simulink model "ClimaCenCfgMgr"  (R2022b)
settings: Solver=FixedStepDiscrete  StartTime=0.0  StopTime=10.0  FixedStep=0.1  RelTol=1e-3
(part 1 of 2)
settings Model: Creator=blue.ge  LastModifiedBy=blue.ge
settings DataIOCC: SaveFormat=StructureWithTime
settings OptimizationCC: DefaultParameterBehavior=Inlined  EnhancedBackFolding=on  PassReuseOutputArgsAs=Individual arguments  ZeroExternalMemoryAtStartup=off  ZeroInternalMemoryAtStartup=off  OptimizeBlockOrder=speed  DifferentSizesBufferReuse=on  OptimizationCustomize=off  EfficientTunableParamExpr=on
settings DebuggingCC: ParameterTunabilityLossMsg=error
settings ModelReferenceCC: ModelReferenceNumInstancesAllowed=Single
settings SFSimCC: MATLABDynamicMemAlloc=off
settings RTWCC: Description=AUTOSAR  SystemTargetFile=autosar.tlc  GenCodeOnly=on  TemplateMakefile=ert_default_tmf
settings CodeAppCC: ForceParamTrailComments=on  IgnoreCustomStorageClasses=off  OperatorAnnotations=on  SimulinkDataObjDesc=on  SFDataObjDesc=on  InsertBlockDesc=on
settings STFCustomTargetCC: TargetLangStandard=C99 (ISO)  MultiwordLength=256  ParMdlRefBuildCompliant=on  CompOptLevelCompliant=on  IncludeMdlTerminateFcn=off  CombineOutputUpdateFcns=on  SuppressErrorStatus=on  ERTCustomFileBanners=on  SupportNonFinite=off  SupportComplex=off  SupportContinuousTime=off  SupportNonInlinedSFcns=off  RemoveResetFunc=on  MATLABClassNameForMDSCustomization=Simulink.SoftwareTarget.ERTCustomization  AutosarCompliant=on  UseToolchainInfoCompliant=on  LookupTableObjectStructAxisOrder=2,1,3,4,...  LUTObjectStructOrderEvenSpacing=Size,Table,Breakpoints  SystemTargetFile=autosar.tlc
settings ERTTargetCC: TargetLangStandard=C99 (ISO)  ConcurrentExecutionCompliant=off  IncludeMdlTerminateFcn=off  SuppressErrorStatus=on  SupportNonFinite=off  SupportComplex=off  ModelStepFunctionPrototypeControlCompliant=off  CPPClassGenCompliant=off  AutosarCompliant=on  LookupTableObjectStructAxisOrder=2,1,3,4,...  LUTObjectStructOrderEvenSpacing=Size,Table,Breakpoints  InlinedParameterPlacement=NonHierarchical  ERTCustomFileTemplate=example_file_process.tlc

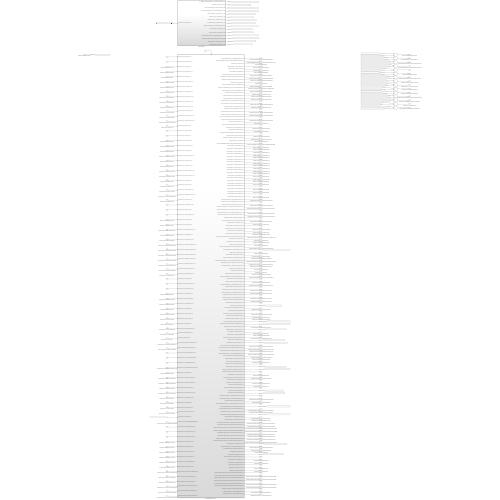
[thumbnail: Simulink's stored preview of the top-level diagram]
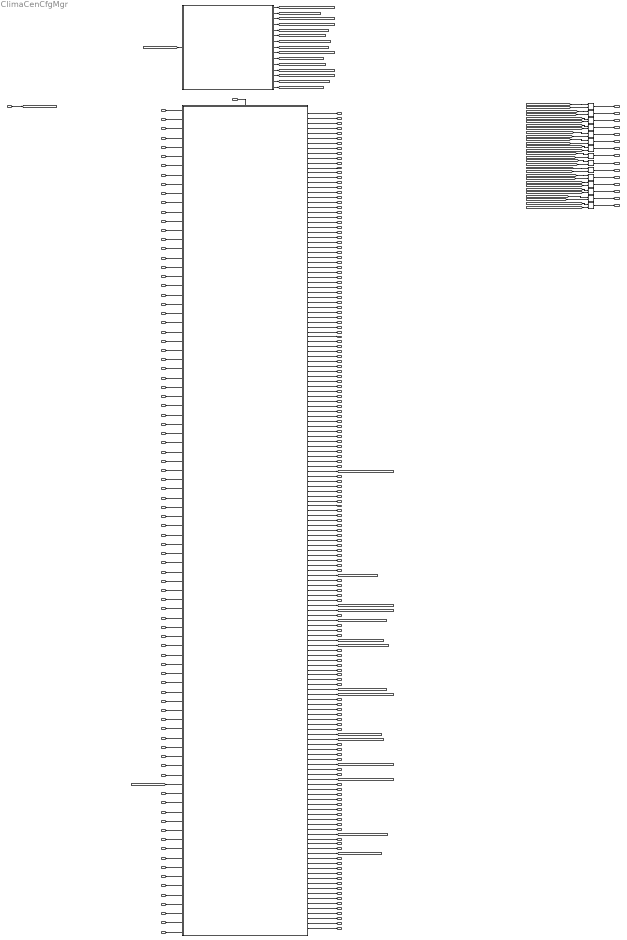
== system ClimaCenCfgMgr ==
  ClimaCenCfgMgr_Runnable : Inport  SampleTime=0.1  OutputFunctionCall=on
  CarCfgForClimaCtrl_CarCfgForRFKEY : Inport  Port=2  OutDataTypeStr=UInt8  PortDimensions=1
  CarCfgForClimaCtrl_CarCfgForAirClng : Inport  Port=3  OutDataTypeStr=Enum: CarCfgForAirClng  PortDimensions=1
  CarCfgForClimaCtrl_CarCfgForAirClnrAdv : Inport  Port=4  OutDataTypeStr=UInt8  PortDimensions=1
  CarCfgForClimaCtrl_CarCfgForAirFrag : Inport  Port=5  OutDataTypeStr=Enum: CarCfgForAirFrag  PortDimensions=1
  CarCfgForClimaCtrl_CarCfgForAirMtrlSnsr : Inport  Port=6  OutDataTypeStr=Enum: CarCfgForAirMtrlSnsrGly  PortDimensions=1
  CarCfgForClimaCtrl_CarCfgForAirQly : Inport  Port=7  OutDataTypeStr=Enum: CarCfgForAirQlyGly  PortDimensions=1
  CarCfgForClimaCtrl_CarCfgForAmntOfSeat : Inport  Port=8  OutDataTypeStr=Enum: CarCfgForAmntOfSeatTyp3  PortDimensions=1
  CarCfgForClimaCtrl_CarCfgForAutDemst : Inport  Port=9  OutDataTypeStr=UInt8  PortDimensions=1
  CarCfgForClimaCtrl_CarCfgForAuxWtrPmp : Inport  Port=10  OutDataTypeStr=Enum: CarCfgForAuxWtrPmp  PortDimensions=1
  CarCfgForClimaCtrl_CarCfgForBypVlvInHvac : Inport  Port=11  OutDataTypeStr=Enum: CarCfgForBypVlvInHvac2  PortDimensions=1
  CarCfgForClimaCtrl_CarCfgForClsVlvInHvac : Inport  Port=12  OutDataTypeStr=Enum: CarCfgForClsVlvInHvac  PortDimensions=1
  CarCfgForClimaCtrl_CarCfgForCmprAcTyp : Inport  Port=13  OutDataTypeStr=UInt8  PortDimensions=1
  CarCfgForClimaCtrl_CarCfgForCmptmtClng : Inport  Port=14  OutDataTypeStr=Enum: CarCfgForCmptmtClng  PortDimensions=1
  CarCfgForClimaCtrl_CarCfgForElecVentAdjmt : Inport  Port=15  OutDataTypeStr=Enum: CarCfgForElecVentAdjmt  PortDimensions=1
  CarCfgForClimaCtrl_CarCfgForExtVentnClimaCtrl : Inport  Port=16  OutDataTypeStr=Enum: CarCfgForExtVentnClimaCtrl  PortDimensions=1
  CarCfgForClimaCtrl_CarCfgForFlcTyp : Inport  Port=17  OutDataTypeStr=Enum: CarCfgForFlcTyp  PortDimensions=1
  CarCfgForClimaCtrl_CarCfgForFoldCSD : Inport  Port=18  OutDataTypeStr=Enum: CarCfgForFoldCSD  PortDimensions=1
  CarCfgForClimaCtrl_CarCfgForFuTyp : Inport  Port=19  OutDataTypeStr=Enum: CarCfgForFuTyp  PortDimensions=1
  CarCfgForClimaCtrl_CarCfgForGlassTyp : Inport  Port=20  OutDataTypeStr=Enum: CarCfgForGlassTyp  PortDimensions=1
  CarCfgForClimaCtrl_CarCfgForHeadRadar : Inport  Port=21  OutDataTypeStr=Enum: HeadRadar  PortDimensions=1
  CarCfgForClimaCtrl_CarCfgForHeatPmp : Inport  Port=22  OutDataTypeStr=Enum: CarCfgForHeatPmpTyp  PortDimensions=1
  CarCfgForClimaCtrl_CarCfgForHeatrDurgDrvg : Inport  Port=23  OutDataTypeStr=Enum: CarCfgForHeatrDurgDrvg  PortDimensions=1
  CarCfgForClimaCtrl_CarCfgForHeatrTyp : Inport  Port=24  OutDataTypeStr=UInt8  PortDimensions=1
  CarCfgForClimaCtrl_CarCfgForHvacFilTyp : Inport  Port=25  OutDataTypeStr=Enum: CarCfgForHvacFilTypGly  PortDimensions=1
  CarCfgForClimaCtrl_CarCfgForHvacFlrSnsrForT : Inport  Port=26  OutDataTypeStr=Enum: CarCfgForHvacFlrSnsrForT  PortDimensions=1
  CarCfgForClimaCtrl_CarCfgForHvacHeatrSnsrT : Inport  Port=27  OutDataTypeStr=Enum: CarCfgForHvacHeatrSnsrT  PortDimensions=1
  CarCfgForClimaCtrl_CarCfgForHvacRe : Inport  Port=28  OutDataTypeStr=UInt8  PortDimensions=1
  CarCfgForClimaCtrl_CarCfgForHvacTyp : Inport  Port=29  OutDataTypeStr=UInt8  PortDimensions=1
  CarCfgForClimaCtrl_CarCfgForLoVoltElecCfg : Inport  Port=30  OutDataTypeStr=Enum: CarCfgForLoVoltElecCfg  PortDimensions=1
  CarCfgForClimaCtrl_CarCfgForNavSysandIntnmap : Inport  Port=31  OutDataTypeStr=Enum: CarCfgForNavSysandIntnmap  PortDimensions=1
  CarCfgForClimaCtrl_CarCfgForNrOfDoor : Inport  Port=32  OutDataTypeStr=Enum: CarCfgForNrOfDoor2  PortDimensions=1
  CarCfgForClimaCtrl_CarCfgForPipeInFuTank : Inport  Port=33  OutDataTypeStr=Enum: CarCfgForPipeInFuTank  PortDimensions=1
  CarCfgForClimaCtrl_CarCfgForPreClng : Inport  Port=34  OutDataTypeStr=Enum: CarCfgForPreClng  PortDimensions=1
  CarCfgForClimaCtrl_CarCfgForPrkgCDSNotAvl : Inport  Port=35  OutDataTypeStr=Enum: CarCfgForPrkgCDSNotAvl  PortDimensions=1
  CarCfgForClimaCtrl_CarCfgForPrkgClima : Inport  Port=36  OutDataTypeStr=Enum: CarCfgForPrkgClima  PortDimensions=1
  CarCfgForClimaCtrl_CarCfgForPrpsnTyp : Inport  Port=37  OutDataTypeStr=Enum: CarCfgForPrpsnTypGly  PortDimensions=1
  CarCfgForClimaCtrl_CarCfgForRemEngStrtPreCdn : Inport  Port=38  OutDataTypeStr=Enum: CarCfgForRemEngStrtPreCdn  PortDimensions=1
  CarCfgForClimaCtrl_CarCfgForRoofHatch : Inport  Port=39  OutDataTypeStr=Enum: CarCfgForRoofHatch  PortDimensions=1
  CarCfgForClimaCtrl_CarCfgForSeatHeatgCal : Inport  Port=40  OutDataTypeStr=Enum: CarCfgForSeatHeatgCal1  PortDimensions=1
  CarCfgForClimaCtrl_CarCfgForSeatHeatgForRowFirst : Inport  Port=41  OutDataTypeStr=Enum: CarCfgForSeatHeatgForRowFirst  PortDimensions=1
  CarCfgForClimaCtrl_CarCfgForSeatHeatgForRowSec : Inport  Port=42  OutDataTypeStr=Enum: CarCfgForSeatHeatgForRowSec  PortDimensions=1
  CarCfgForClimaCtrl_CarCfgForSeatVentnForRowFirst : Inport  Port=43  OutDataTypeStr=Enum: CarCfgForSeatVentnForRowFirst1  PortDimensions=1
  CarCfgForClimaCtrl_CarCfgForSeatVentnForRowSec : Inport  Port=44  OutDataTypeStr=Enum: CarCfgForSeatVentnForRowSecGly  PortDimensions=1
  CarCfgForClimaCtrl_CarCfgForSecRowVentnCenter : Inport  Port=45  OutDataTypeStr=Enum: CarCfgForSecRowVentnCenter  PortDimensions=1
  CarCfgForClimaCtrl_CarCfgForSecRowVentnSide : Inport  Port=46  OutDataTypeStr=Enum: CarCfgForSecRowVentnSide  PortDimensions=1
  CarCfgForClimaCtrl_CarCfgForSmallFlowCyc : Inport  Port=47  OutDataTypeStr=Enum: CarCfgForSmallFlowCyc  PortDimensions=1
  CarCfgForClimaCtrl_CarCfgForSmkSnsr : Inport  Port=48  OutDataTypeStr=Enum: CarCfgForSmkSnsr  PortDimensions=1
  CarCfgForClimaCtrl_CarCfgForSnsrForCmptmtT : Inport  Port=49  OutDataTypeStr=Enum: CarCfgForSnsrForCmptmtT  PortDimensions=1
  CarCfgForClimaCtrl_CarCfgForSnsrHumPrsnt : Inport  Port=50  OutDataTypeStr=Enum: CarCfgForSnsrHumPrsnt  PortDimensions=1
  CarCfgForClimaCtrl_CarCfgForStartDevice : Inport  Port=51  OutDataTypeStr=Enum: StartDevice  PortDimensions=1
  CarCfgForClimaCtrl_CarCfgForSteerWhlHeatg : Inport  Port=52  OutDataTypeStr=UInt8  PortDimensions=1
  CarCfgForClimaCtrl_CarCfgForSteerWhlPosn : Inport  Port=53  OutDataTypeStr=Enum: CarCfgForSteerWhlPosn  PortDimensions=1
  CarCfgForClimaCtrl_CarCfgForSunSnsr : Inport  Port=54  OutDataTypeStr=Enum: CarCfgForSunSnsr  PortDimensions=1
  CarCfgForClimaCtrl_CarCfgForTelmNodTyp : Inport  Port=55  OutDataTypeStr=Enum: CarCfgForTelmNodTyp  PortDimensions=1
  CarCfgForClimaCtrl_CarCfgForThermMngt : Inport  Port=56  OutDataTypeStr=Enum: CarCfgForThermMngt  PortDimensions=1
  CarCfgForClimaCtrl_CarCfgForVehTyp : Inport  Port=57  OutDataTypeStr=Enum: CarCfgForVehTyp3  PortDimensions=1
  CarCfgForClimaCtrl_CarCfgForVgaMotorExistSts : Inport  Port=58  OutDataTypeStr=CarCfgForVgaMotorExistSts  PortDimensions=1
  CarCfgForClimaCtrl_CarCfgForWindHeatd : Inport  Port=59  OutDataTypeStr=Enum: CarCfgForWindHeatd  PortDimensions=1
  CooltVlvOutpTyp_CooltVlvOutpTyp : Inport  Port=60  OutDataTypeStr=Enum: CooltVlvOutpTyp  PortDimensions=1
  HvacCfgForClimaCtrl_HvacCfgForHvacFrontBlowerType : Inport  Port=61  OutDataTypeStr=HvacCfgForHvacFrontBlowerType  PortDimensions=1
  HvacCfgForClimaCtrl_HvacCfgForHvacMiscExistSts : Inport  Port=62  OutDataTypeStr=HvacCfgForHvacMiscExistSts  PortDimensions=1
  HvacCfgForClimaCtrl_HvacCfgForHvacMotorExistSts : Inport  Port=63  OutDataTypeStr=HvacCfgForHvacMotorExistSts  PortDimensions=1
  HvacCfgForClimaCtrl_HvacCfgForHvacSensorExistSts : Inport  Port=64  OutDataTypeStr=HvacCfgForHvacSensorExistSts  PortDimensions=1
  CarCfgForClimaCtrl_CarCfgFor3RDROWSEATHEAT : Inport  Port=65  OutDataTypeStr=UInt8  PortDimensions=1
  CarCfgForClimaCtrl_CarCfgFor3RDROWSEATVENTILATED : Inport  Port=66  OutDataTypeStr=UInt8  PortDimensions=1
  CarCfgForClimaCtrl_CarCfgForBRAND : Inport  Port=67  OutDataTypeStr=UInt8  PortDimensions=1
  CarCfgForClimaCtrl_CarCfgForCabinOxgenSupply : Inport  Port=68  OutDataTypeStr=UInt8  PortDimensions=1
  CarCfgForClimaCtrl_CarCfgForElectricLiftingModule : Inport  Port=69  OutDataTypeStr=UInt8  PortDimensions=1
  CarCfgForClimaCtrl_CarCfgForFloatingDriving : Inport  Port=70  OutDataTypeStr=UInt8  PortDimensions=1
  CarCfgForClimaCtrl_CarCfgForInteriorViewCamera : Inport  Port=71  OutDataTypeStr=Enum: CarCfgForInteriorViewCamera  PortDimensions=1
  CarCfgForClimaCtrl_CarCfgForLifeDetection : Inport  Port=72  OutDataTypeStr=Enum: LifeDetection  PortDimensions=1
  CarCfgForClimaCtrl_CarCfgForMARKET : Inport  Port=73  OutDataTypeStr=UInt8  PortDimensions=1
  CarCfgForClimaCtrl_CarCfgForPowerSeats : Inport  Port=74  OutDataTypeStr=Enum: CarCfgForPowerSeats  PortDimensions=1
  CarCfgForClimaCtrl_CarCfgForPTCInFrontHVAC : Inport  Port=75  OutDataTypeStr=UInt8  PortDimensions=1
  CarCfgForClimaCtrl_CarCfgForTEMPEREDSTORAGEBOX : Inport  Port=76  OutDataTypeStr=UInt8  PortDimensions=1
  CarCfgForClimaCtrl_CarCfgForVentLiftMotorExiSts : Inport  Port=77  OutDataTypeStr=UInt32  PortDimensions=1
  CarCfgForClimaCtrl_CarCfgForWadingAssistSystem : Inport  Port=78  OutDataTypeStr=UInt8  PortDimensions=1
  CarCfgForClimaCtrl_CarCfgWindFollowHumanMov : Inport  Port=79  OutDataTypeStr=UInt8  PortDimensions=1
  CenCfgMgrForCompile_CarCfgForAirClnrAdv : Inport  Port=80  OutDataTypeStr=Enum: CarCfgForAirClnrAdv  PortDimensions=1
  CenCfgMgrForCompile_CarCfgForAutDemst : Inport  Port=81  OutDataTypeStr=Enum: CarCfgForAutDemst  PortDimensions=1
  CenCfgMgrForCompile_CarCfgForCmprAcTyp3 : Inport  Port=82  OutDataTypeStr=Enum: CarCfgForCmprAcTyp3  PortDimensions=1
  CenCfgMgrForCompile_CarCfgForHeatrTypGly : Inport  Port=83  OutDataTypeStr=Enum: CarCfgForHeatrTypGly  PortDimensions=1
  CenCfgMgrForCompile_CarCfgForSteerWhlHeatg : Inport  Port=84  OutDataTypeStr=Enum: CarCfgForSteerWhlHeatg  PortDimensions=1
  HvacCfgForClimaCtrl_HvacCfgForAQSTypes : Inport  Port=85  OutDataTypeStr=UInt8  PortDimensions=1
  HvacCfgForClimaCtrl_HvacCfgForHvacLowSideRETXVESts : Inport  Port=86  OutDataTypeStr=UInt8  PortDimensions=1
  HvacCfgForClimaCtrl_HvacCfgForHvacReBlowerType : Inport  Port=87  OutDataTypeStr=UInt8  PortDimensions=1
  HvacCfgForClimaCtrl_HvacCfgForHvacReVentPstn : Inport  Port=88  OutDataTypeStr=UInt8  PortDimensions=1
  HvacCfgForClimaCtrl_HvacCfgForHvacSnsrRCmptmtESts : Inport  Port=89  OutDataTypeStr=UInt8  PortDimensions=1
  HvacCfgForClimaCtrl_HvacCfgForHvacSnsrReHexESts : Inport  Port=90  OutDataTypeStr=UInt8  PortDimensions=1
  HvacCfgReqVersionReplace_HvacCfgReqVersionReplace : Inport  Port=91  OutDataTypeStr=UInt8  PortDimensions=1
  ClimaCenCfgMgr_Init : Subsystem
  ClimaCenCfgMgr_Runnable_sys : Subsystem
  ClimaCfgForAirMtrlSnsrPrsnt1_ClimaCfgForAirMtrlSnsrPrsnt1_merge : Merge
  ClimaCfgForFlcTyp_ClimaCfgForFlcTyp_merge : Merge
  ClimaCfgForForLoVoltElecCfg_ClimaCfgForForLoVoltElecCfg_merge : Merge
  ClimaCfgForForRemEngStrtPreCdn_ClimaCfgForForRemEngStrtPreCdn_merge : Merge
  ClimaCfgForFuHeatrTyp1_ClimaCfgForFuHeatrTyp1_merge : Merge
  ClimaCfgForHeatrTyp1_ClimaCfgForHeatrTyp1_merge : Merge
  ClimaCfgForHvHeatrTyp1_ClimaCfgForHvHeatrTyp1_merge : Merge
  ClimaCfgForHvacFilVrnt1_ClimaCfgForHvacFilVrnt1_merge : Merge
  ClimaCfgForNavSysandIntnmap_ClimaCfgForNavSysandIntnmap_merge : Merge
  ClimaCfgForPrpsnTyp_ClimaCfgForPrpsnTyp_merge : Merge
  ClimaCfgForRoofHatch_ClimaCfgForRoofHatch_merge : Merge
  ClimaCfgForSeatHeatgForRowSec_ClimaCfgForSeatHeatgForRowSec_merge : Merge
  ClimaCfgForSeatVentnForRowSec_ClimaCfgForSeatVentnForRowSec_merge : Merge
  ClimaCfgForStatrtDevice_ClimaCfgForStartDevice_merge : Merge
  ClimaCfgForSunSnsr1_ClimaCfgForSunSnsr1_merge : Merge
  ClimaCfgForAirMtrlSnsrPrsnt1_ClimaCfgForAirMtrlSnsrPrsnt1 : Outport  OutDataTypeStr=Enum: CarCfgForAirMtrlSnsrGly  PortDimensions=1
  ClimaCfgForFlcTyp_ClimaCfgForFlcTyp : Outport  Port=2  OutDataTypeStr=Enum: CarCfgForFlcTyp  PortDimensions=1
  ClimaCfgForForLoVoltElecCfg_ClimaCfgForForLoVoltElecCfg : Outport  Port=3  OutDataTypeStr=Enum: CarCfgForLoVoltElecCfg  PortDimensions=1
  ClimaCfgForForRemEngStrtPreCdn_ClimaCfgForForRemEngStrtPreCdn : Outport  Port=4  OutDataTypeStr=Enum: CarCfgForRemEngStrtPreCdn  PortDimensions=1
  ClimaCfgForFuHeatrTyp1_ClimaCfgForFuHeatrTyp1 : Outport  Port=5  OutDataTypeStr=Enum: ClimaCfgForFuHeatrTyp1  PortDimensions=1
  ClimaCfgForHeatrTyp1_ClimaCfgForHeatrTyp1 : Outport  Port=6  OutDataTypeStr=Enum: ClimaCfgForHeatrTyp1  PortDimensions=1
  ClimaCfgForHvacFilVrnt1_ClimaCfgForHvacFilVrnt1 : Outport  Port=7  OutDataTypeStr=Enum: CarCfgForHvacFilTypGly  PortDimensions=1
  ClimaCfgForHvHeatrTyp1_ClimaCfgForHvHeatrTyp1 : Outport  Port=8  OutDataTypeStr=Enum: ClimaCfgForHvHeatrTyp1  PortDimensions=1
  ClimaCfgForNavSysandIntnmap_ClimaCfgForNavSysandIntnmap : Outport  Port=9  OutDataTypeStr=Enum: CarCfgForNavSysandIntnmap  PortDimensions=1
  ClimaCfgForPrpsnTyp_ClimaCfgForPrpsnTyp : Outport  Port=10  OutDataTypeStr=Enum: CarCfgForPrpsnTypGly  PortDimensions=1
  ClimaCfgForRoofHatch_ClimaCfgForRoofHatch : Outport  Port=11  OutDataTypeStr=Enum: CarCfgForRoofHatch  PortDimensions=1
  ClimaCfgForSeatHeatgForRowSec_ClimaCfgForSeatHeatgForRowSec : Outport  Port=12  OutDataTypeStr=Enum: CarCfgForSeatHeatgForRowSecGly  PortDimensions=1
  ClimaCfgForSeatVentnForRowSec_ClimaCfgForSeatVentnForRowSec : Outport  Port=13  OutDataTypeStr=Enum: CarCfgForSeatVentnForRowSecGly  PortDimensions=1
  ClimaCfgForStatrtDevice_ClimaCfgForStartDevice : Outport  Port=14  OutDataTypeStr=Enum: StartDevice  PortDimensions=1
  ClimaCfgForSunSnsr1_ClimaCfgForSunSnsr1 : Outport  Port=15  OutDataTypeStr=Enum: CarCfgForSunSnsr  PortDimensions=1
  ClimaCfgFor3RDROWSEATHEAT_ClimaCfgFor3RDROWSEATHEAT : Outport  Port=16  OutDataTypeStr=UInt8  PortDimensions=1
  ClimaCfgFor3RDROWSEATVENTILATED_ClimaCfgFor3RDROWSEATVENTILATED : Outport  Port=17  OutDataTypeStr=UInt8  PortDimensions=1
  ClimaCfgForAAC_ClimaCfgForAAC : Outport  Port=18  OutDataTypeStr=Enum: CarCfgForAirClng  PortDimensions=1
  ClimaCfgForAQSTypes_ClimaCfgForAQSTypes : Outport  Port=19  OutDataTypeStr=UInt8  PortDimensions=1
  ClimaCfgForAutDemst_ClimaCfgForAutDemst : Outport  Port=20  OutDataTypeStr=Boolean  PortDimensions=1
  ClimaCfgForBRAND_ClimaCfgForBRAND : Outport  Port=21  OutDataTypeStr=Enum: ClimaCfgForBRAND  PortDimensions=1
  ClimaCfgForCabinOxgenSupply_ClimaCfgForCabinOxgenSupply : Outport  Port=22  OutDataTypeStr=Enum: CabinOxgenSupply  PortDimensions=1
  ClimaCfgForDrvrSeatVentWthSmf2_ClimaCfgForDrvrSeatVentWthSmf2 : Outport  Port=23  OutDataTypeStr=UInt8  PortDimensions=1
  ClimaCfgForElectricLiftingModule_ClimaCfgForElectricLiftingModule : Outport  Port=24  OutDataTypeStr=Enum: ElcTrLFtngMdle  PortDimensions=1
  ClimaCfgForErev1_ClimaCfgForErev1 : Outport  Port=25  OutDataTypeStr=Boolean  PortDimensions=1
  ClimaCfgForFLOATINGDRVING_ClimaCfgForFLOATINGDRVING : Outport  Port=26  OutDataTypeStr=Enum: FLOATINGDRVING  PortDimensions=1
  ClimaCfgForHvacLowSideRETXVESts_ClimaCfgForHvacLowSideRETXVESts : Outport  Port=27  OutDataTypeStr=Enum: ClimaCfgForHvacLowSideRETXVESts  PortDimensions=1
  ClimaCfgForHvacReBlowerType_ClimaCfgForHvacReBlowerType : Outport  Port=28  OutDataTypeStr=Enum: ClimaCfgForHvacReBlowerType  PortDimensions=1
  ClimaCfgForHvacReTmpZn_ClimaCfgForHvacReTmpZn : Outport  Port=29  OutDataTypeStr=UInt8  PortDimensions=1
  ClimaCfgForHvacReVentPstn_ClimaCfgForRowSecAtFlrLeRe : Outport  Port=30  OutDataTypeStr=UInt8  PortDimensions=1
  ClimaCfgForHvacReVentPstn_ClimaCfgForRowSecAtFlrRiRe : Outport  Port=31  OutDataTypeStr=UInt8  PortDimensions=1
  ClimaCfgForHvacReVentPstn_ClimaCfgForRowSecAtVentSideTopLe : Outport  Port=32  OutDataTypeStr=UInt8  PortDimensions=1
  ClimaCfgForHvacReVentPstn_ClimaCfgForRowSecAtVentSideTopRi : Outport  Port=33  OutDataTypeStr=UInt8  PortDimensions=1
  ClimaCfgForHvacReVentPstn_ClimaCfgForRowThrdAtFlrLe : Outport  Port=34  OutDataTypeStr=UInt8  PortDimensions=1
  ClimaCfgForHvacReVentPstn_ClimaCfgForRowThrdAtFlrRi : Outport  Port=35  OutDataTypeStr=UInt8  PortDimensions=1
  ClimaCfgForHvacReVentPstn_ClimaCfgForRowThrdAtVentSideTopLe : Outport  Port=36  OutDataTypeStr=UInt8  PortDimensions=1
  ClimaCfgForHvacReVentPstn_ClimaCfgForRowThrdAtVentSideTopRi : Outport  Port=37  OutDataTypeStr=UInt8  PortDimensions=1
  ClimaCfgForHvacSnsrRCmptmtESts_ClimaCfgForHvacSnsrRCmptmtESts : Outport  Port=38  OutDataTypeStr=Enum: ClimaCfgForHvacSnsrRCmptmtESts  PortDimensions=1
  ClimaCfgForHvacSnsrReHexESts_ClimaCfgForHvacSnsrReHexESts : Outport  Port=39  OutDataTypeStr=Enum: ClimaCfgForHvacSnsrReHexESts  PortDimensions=1
  ClimaCfgForHvacTyp_ClimaCfgForHvacTyp : Outport  Port=40  OutDataTypeStr=Enum: CarCfgForHvacTyp2  PortDimensions=1
  ClimaCfgForInteriorViewCamera_ClimaCfgForInteriorViewCamera : Outport  Port=41  OutDataTypeStr=Enum: CarCfgForInteriorViewCamera  PortDimensions=1
  ClimaCfgForLifeDetection_ClimaCfgForLifeDetection : Outport  Port=42  OutDataTypeStr=Enum: LifeDetection  PortDimensions=1
  ClimaCfgForMARKET_ClimaCfgForMARKET : Outport  Port=43  OutDataTypeStr=UInt8  PortDimensions=1
  ClimaCfgForPassSeatVentWthSmf2_ClimaCfgForPassSeatVentWthSmf2 : Outport  Port=44  OutDataTypeStr=UInt8  PortDimensions=1
  ClimaCfgForPowerSeats_ClimaCfgForPowerSeats : Outport  Port=45  OutDataTypeStr=Enum: CarCfgForPowerSeats  PortDimensions=1
  ClimaCfgForPTCInFrontHVAC_ClimaCfgForPTCInFrontHVAC : Outport  Port=46  OutDataTypeStr=Enum: FrontHeaterExchanger  PortDimensions=1
  ClimaCfgForRFKEY_ClimaCfgForRFKEY : Outport  Port=47  OutDataTypeStr=Enum: RFKEY  PortDimensions=1
  ClimaCfgForTEMPEREDSTORAGEBOX_ClimaCfgForTEMPEREDSTORAGEBOX : Outport  Port=48  OutDataTypeStr=Enum: TEMPEREDSTORAGEBOX  PortDimensions=1
  ClimaCfgForVgaMotor1_ClimaCfgForVgaMotor1 : Outport  Port=49  OutDataTypeStr=Boolean  PortDimensions=1
  ClimaCfgForVgaMotor10_ClimaCfgForVgaMotor10 : Outport  Port=50  OutDataTypeStr=Boolean  PortDimensions=1
  ClimaCfgForVgaMotor11_ClimaCfgForVgaMotor11 : Outport  Port=51  OutDataTypeStr=Boolean  PortDimensions=1
  ClimaCfgForVgaMotor12_ClimaCfgForVgaMotor12 : Outport  Port=52  OutDataTypeStr=Boolean  PortDimensions=1
  ClimaCfgForVgaMotor13_ClimaCfgForVgaMotor13 : Outport  Port=53  OutDataTypeStr=Boolean  PortDimensions=1
  ClimaCfgForVgaMotor14_ClimaCfgForVgaMotor14 : Outport  Port=54  OutDataTypeStr=Boolean  PortDimensions=1
  ClimaCfgForVgaMotor15_ClimaCfgForVgaMotor15 : Outport  Port=55  OutDataTypeStr=Boolean  PortDimensions=1
  ClimaCfgForVgaMotor16_ClimaCfgForVgaMotor16 : Outport  Port=56  OutDataTypeStr=Boolean  PortDimensions=1
  ClimaCfgForVgaMotor17_ClimaCfgForVgaMotor17 : Outport  Port=57  OutDataTypeStr=Boolean  PortDimensions=1
  ClimaCfgForVgaMotor18_ClimaCfgForVgaMotor18 : Outport  Port=58  OutDataTypeStr=Boolean  PortDimensions=1
  ClimaCfgForVgaMotor19_ClimaCfgForVgaMotor19 : Outport  Port=59  OutDataTypeStr=Boolean  PortDimensions=1
  ClimaCfgForVgaMotor2_ClimaCfgForVgaMotor2 : Outport  Port=60  OutDataTypeStr=Boolean  PortDimensions=1
  ClimaCfgForVgaMotor20_ClimaCfgForVgaMotor20 : Outport  Port=61  OutDataTypeStr=Boolean  PortDimensions=1
  ClimaCfgForVgaMotor3_ClimaCfgForVgaMotor3 : Outport  Port=62  OutDataTypeStr=Boolean  PortDimensions=1
  ClimaCfgForVgaMotor4_ClimaCfgForVgaMotor4 : Outport  Port=63  OutDataTypeStr=Boolean  PortDimensions=1
  ClimaCfgForVgaMotor5_ClimaCfgForVgaMotor5 : Outport  Port=64  OutDataTypeStr=Boolean  PortDimensions=1
  ClimaCfgForVgaMotor6_ClimaCfgForVgaMotor6 : Outport  Port=65  OutDataTypeStr=Boolean  PortDimensions=1
  ClimaCfgForVgaMotor7_ClimaCfgForVgaMotor7 : Outport  Port=66  OutDataTypeStr=Boolean  PortDimensions=1
  ClimaCfgForVgaMotor8_ClimaCfgForVgaMotor8 : Outport  Port=67  OutDataTypeStr=Boolean  PortDimensions=1
  ClimaCfgForVgaMotor9_ClimaCfgForVgaMotor9 : Outport  Port=68  OutDataTypeStr=Boolean  PortDimensions=1
  ClimaCfgForWadingAssistSystem_ClimaCfgForWadingAssistSystem : Outport  Port=69  OutDataTypeStr=Enum: WadingAssistSystem  PortDimensions=1
  ClimaCfgHvacForFrntLeTempActr_ClimaCfgHvacForFrntLeTempActr : Outport  Port=70  OutDataTypeStr=Boolean  PortDimensions=1
  ClimaCfgHvacForFrntRiTempActr_ClimaCfgHvacForFrntRiTempActr : Outport  Port=71  OutDataTypeStr=Boolean  PortDimensions=1
  ClimaCfgHvacFrntForRowSecForActrTLe_ClimaCfgHvacFrntForRowSecForActrTLe : Outport  Port=72  OutDataTypeStr=Boolean  PortDimensions=1
  ClimaCfgHvacFrntForRowSecForActrTRi_ClimaCfgHvacFrntForRowSecForActrTRi : Outport  Port=73  OutDataTypeStr=Boolean  PortDimensions=1
  ClimaCfgHvacReForRowSecForActrTLe_ClimaCfgHvacReForRowSecForActrTLe : Outport  Port=74  OutDataTypeStr=UInt8  PortDimensions=1
  ClimaCfgHvacReForRowSecForActrTRi_ClimaCfgHvacReForRowSecForActrTRi : Outport  Port=75  OutDataTypeStr=UInt8  PortDimensions=1
  ClimaCfgReqVersionReplace_ClimaCfgReqVersionReplace : Outport  Port=76  OutDataTypeStr=UInt8  PortDimensions=1
  ClimaCfgWindFollowHumanMov_ClimaCfgWindFollowHumanMov : Outport  Port=77  OutDataTypeStr=Enum: WndFllwngHmnMvmntFnctn  PortDimensions=1
  ClimaCfgForActrModVrnt1_ClimaCfgForActrInd1 : Outport  Port=78  OutDataTypeStr=Enum: ClimaCfgForActrInd1  PortDimensions=1
  ClimaCfgForActrModVrnt1_ClimaCfgForActrModVrnt1 : Outport  Port=79  OutDataTypeStr=Enum: ClimaCfgForActrModVrnt1  PortDimensions=1
  ClimaCfgForActrModVrnt1_ClimaCfgForActrModVrntCen : Outport  Port=80  OutDataTypeStr=Enum: ClimaCfgForActrModVrntCen  PortDimensions=1
  ClimaCfgForActrModVrnt2_ClimaCfgForActrInd2 : Outport  Port=81  OutDataTypeStr=Enum: ClimaCfgForActrInd2  PortDimensions=1
  ClimaCfgForActrModVrnt2_ClimaCfgForActrModVrnt2 : Outport  Port=82  OutDataTypeStr=Enum: ClimaCfgForActrModVrnt2  PortDimensions=1
  ClimaCfgForActrRecircAndOsaCmnPrsnt1_ClimaCfgForActrRecircAndOsaCmnPrsnt1 : Outport  Port=83  OutDataTypeStr=Enum: ClimaCfgForActrRecircAndOsaCmnPrsnt1  PortDimensions=1
  ClimaCfgForAirClng1_ClimaCfgForAirClng1 : Outport  Port=84  OutDataTypeStr=Boolean  PortDimensions=1
  ClimaCfgForAirClnrAdv1_ClimaCfgForAirClnrAdv1 : Outport  Port=85  OutDataTypeStr=Boolean  PortDimensions=1
  ClimaCfgForAirFrag_ClimaCfgForAirFrag : Outport  Port=86  OutDataTypeStr=Boolean  PortDimensions=1
  ClimaCfgForAirMtrlSnsrAPMAPrsnt1_ClimaCfgForAirMtrlSnsrAPMAPrsnt1 : Outport  Port=87  OutDataTypeStr=Boolean  PortDimensions=1
  ClimaCfgForAirQly1_ClimaCfgForAirQly1 : Outport  Port=88  OutDataTypeStr=Boolean  PortDimensions=1
  ClimaCfgForAmntOfSeat1_ClimaCfgForAmntOfSeat1 : Outport  Port=89  OutDataTypeStr=Enum: ClimaCfgForAmntOfSeat1  PortDimensions=1
  ClimaCfgForAmntOfSeatRow1_ClimaCfgForAmntOfSeatRow1 : Outport  Port=90  OutDataTypeStr=Enum: ClimaCfgForAmntOfSeatRow1  PortDimensions=1
  ClimaCfgForBlowrInRowSecVentSidePrsnt1_ClimaCfgForBlowrInRowSecVentSidePrsnt1 : Outport  Port=91  OutDataTypeStr=Boolean  PortDimensions=1
  ClimaCfgForBypVlvInHvacExtCtrl1_ClimaCfgForBypVlvInHvacExtCtrl1 : Outport  Port=92  OutDataTypeStr=Boolean  PortDimensions=1
  ClimaCfgForBypVlvInHvacPrsnt1_ClimaCfgForBypVlvInHvacPrsnt1 : Outport  Port=93  OutDataTypeStr=Boolean  PortDimensions=1
  ClimaCfgForCarTyp1_ClimaCfgForCarTyp1 : Outport  Port=94  OutDataTypeStr=Enum: ClimaCfgForCarTyp1  PortDimensions=1
  ClimaCfgForCds1_ClimaCfgForCds1 : Outport  Port=95  OutDataTypeStr=Boolean  PortDimensions=1
  ClimaCfgForClimaCtrlMan1_ClimaCfgForClimaCtrlMan1 : Outport  Port=96  OutDataTypeStr=Boolean  PortDimensions=1
  ClimaCfgForClimaCtrlSwtModlPrsnt_ClimaCfgForClimaCtrlSwtModlPrsnt : Outport  Port=97  OutDataTypeStr=Boolean  PortDimensions=1
  ClimaCfgForCmptmtClng_ClimaCfgForCmptmtClng : Outport  Port=98  OutDataTypeStr=Boolean  PortDimensions=1
  ClimaCfgForCmptmtSnsr1_ClimaCfgForCmptmtSnsr1 : Outport  Port=99  OutDataTypeStr=Enum: ClimaCfgForCmptmtSnsr1  PortDimensions=1
  ClimaCfgForComIfGenn2ForTelm1_ClimaCfgForComIfGenn2ForTelm1 : Outport  Port=100  OutDataTypeStr=Boolean  PortDimensions=1
  ClimaCfgForCoolgPreCdn1_ClimaCfgForCoolgPreCdn1 : Outport  Port=101  OutDataTypeStr=Enum: ClimaCfgForCoolgPreCdn1  PortDimensions=1
  ClimaCfgForCooltCircForClima1_ClimaCfgForCooltCircForClima1 : Outport  Port=102  OutDataTypeStr=Boolean  PortDimensions=1
  ClimaCfgForCpilHeatrElecPrsnt1_ClimaCfgForCpilHeatrElecPrsnt1 : Outport  Port=103  OutDataTypeStr=Boolean  PortDimensions=1
  ClimaCfgForEcuCooltVlvOutp1_ClimaCfgForEcuCooltVlvOutp1 : Outport  Port=104  OutDataTypeStr=Enum: ClimaCfgForEcuCooltVlvOutp1  PortDimensions=1
  ClimaCfgForEngCooltPmpElec1_ClimaCfgForEngCooltPmpElec1 : Outport  Port=105  OutDataTypeStr=Boolean  PortDimensions=1
  ClimaCfgForExtVentnClimaCtrl_ClimaCfgForExtVentnClimaCtrl : Outport  Port=106  OutDataTypeStr=Enum: CarCfgForExtVentnClimaCtrl  PortDimensions=1
  ClimaCfgForFanPotiCtrld1_ClimaCfgForFanPotiCtrld1 : Outport  Port=107  OutDataTypeStr=Boolean  PortDimensions=1
  ClimaCfgForFlrSnsrInHvac1_ClimaCfgForFlrSnsrInHvac1 : Outport  Port=108  OutDataTypeStr=Boolean  PortDimensions=1
  ClimaCfgForFoldCSD_ClimaCfgForFoldCSD : Outport  Port=109  OutDataTypeStr=Boolean  PortDimensions=1
  ClimaCfgForForElecVentAdjmt_ClimaCfgForForElecVentAdjmt : Outport  Port=110  OutDataTypeStr=Boolean  PortDimensions=1
  ClimaCfgForForHeatPmp_ClimaCfgForForHeatPmp : Outport  Port=111  OutDataTypeStr=Enum: CarCfgForHeatPmpTyp  PortDimensions=1
  ClimaCfgForForHeatPmpGly_ClimaCfgForForHeatPmp : Outport  Port=112  OutDataTypeStr=Enum: CarCfgForHeatPmpTyp  PortDimensions=1
  ClimaCfgForFuHeatrFuLine1_ClimaCfgForFuHeatrFuLine1 : Outport  Port=113  OutDataTypeStr=Enum: ClimaCfgForFuHeatrFuLine1  PortDimensions=1
  ClimaCfgForGlassTyp1_ClimaCfgForGlassTyp1 : Outport  Port=114  OutDataTypeStr=Enum: ClimaCfgForGlassTyp1  PortDimensions=1
  ClimaCfgForHeadRadar_ClimaCfgForHeadRadar : Outport  Port=115  OutDataTypeStr=Enum: HeadRadar  PortDimensions=1
  ClimaCfgForHeatgPreCdnAvl1_ClimaCfgForHeatgPreCdnAvl1 : Outport  Port=116  OutDataTypeStr=Boolean  PortDimensions=1
  ClimaCfgForHvacFrontBlowerType_ClimaCfgForHvacFrontBlowerType : Outport  Port=117  OutDataTypeStr=Enum: ClimaCfgForHvacFrontBlowerType  PortDimensions=1
  ClimaCfgForHvacHeatrElecFrntPrsnt1_ClimaCfgForHvacHeatrElecFrntPrsnt1 : Outport  Port=118  OutDataTypeStr=Boolean  PortDimensions=1
  ClimaCfgForHvacHeatrSnsrTPrsnt1_ClimaCfgForHvacHeatrSnsrTPrsnt1 : Outport  Port=119  OutDataTypeStr=Boolean  PortDimensions=1
  ClimaCfgForHvacManWithPartRecirc2_ClimaCfgForHvacManWithPartRecirc2 : Outport  Port=120  OutDataTypeStr=Boolean  PortDimensions=1
  ClimaCfgForHvacMiscExistSts_ClimaCfgForHvacMiscExistSts : Outport  Port=121  OutDataTypeStr=Boolean  PortDimensions=1
  ClimaCfgForHvacRePrsnt1_ClimaCfgForHvacRePrsnt1 : Outport  Port=122  OutDataTypeStr=Enum: CarCfgForHvacRe  PortDimensions=1
  ClimaCfgForHvacReTyp1_ClimaCfgForHvacReTyp1 : Outport  Port=123  OutDataTypeStr=Enum: ClimaCfgForHvacReTyp1  PortDimensions=1
  ClimaCfgForHvacSnsrCfg1_ClimaCfgForHvacSnsrCfg1 : Outport  Port=124  OutDataTypeStr=Bus: HvacSnsrCfg1  PortDimensions=1  BusOutputAsStruct=on
  ClimaCfgForNrOfAirDistbnSetg1_ClimaCfgForNrOfAirDistbnSetg1 : Outport  Port=125  OutDataTypeStr=Enum: ClimaCfgForNrOfAirDistbnSetg1  PortDimensions=1
  ClimaCfgForNrOfDoor1_ClimaCfgForNrOfDoor1 : Outport  Port=126  OutDataTypeStr=Enum: ClimaCfgForNrOfDoor1  PortDimensions=1
  ClimaCfgForNrOfFanLvlSetg1_ClimaCfgForNrOfFanLvlSetg1 : Outport  Port=127  OutDataTypeStr=Enum: ClimaCfgForNrOfFanLvlSetg1  PortDimensions=1
  ClimaCfgForNrOfTSetg1_ClimaCfgForNrOfTSetg1 : Outport  Port=128  OutDataTypeStr=Enum: ClimaCfgForNrOfTSetg1  PortDimensions=1
  ClimaCfgForPostCdnAvl1_ClimaCfgForPostCdnAvl1 : Outport  Port=129  OutDataTypeStr=Boolean  PortDimensions=1
  ClimaCfgForPreClng1_ClimaCfgForPreClng1 : Outport  Port=130  OutDataTypeStr=Boolean  PortDimensions=1
  ClimaCfgForPrkgCDSNotAvl_ClimaCfgForPrkgCDSNotAvl : Outport  Port=131  OutDataTypeStr=Boolean  PortDimensions=1
  ClimaCfgForRowSecVentCentrVrnt1_ClimaCfgForRowSecVentCentrVrnt1 : Outport  Port=132  OutDataTypeStr=Enum: ClimaCfgForRowSecVentCentrVrnt1  PortDimensions=1
  ClimaCfgForRowSecVentSidePrsnt1_ClimaCfgForRowSecVentSidePrsnt1 : Outport  Port=133  OutDataTypeStr=Boolean  PortDimensions=1
  ClimaCfgForSeatHeatgCal1_ClimaCfgForSeatHeatgCal1 : Outport  Port=134  OutDataTypeStr=Enum: CarCfgForSeatHeatgCal1  PortDimensions=1
  ClimaCfgForSeatHeatgForRowFirstPrsnt1_ClimaCfgForSeatHeatgForRowFirstPrsnt1 : Outport  Port=135  OutDataTypeStr=Boolean  PortDimensions=1
  ClimaCfgForSeatHeatrElmDualPrsnt1_ClimaCfgForSeatHeatrElmDualPrsnt1 : Outport  Port=136  OutDataTypeStr=Boolean  PortDimensions=1
  ClimaCfgForSeatVentnForRowFirst_ClimaCfgForSeatVentnForRowFirst : Outport  Port=137  OutDataTypeStr=Enum: CarCfgForSeatVentnForRowFirst1  PortDimensions=1
  ClimaCfgForSmkSnsrPrsnt1_ClimaCfgForSmkSnsrPrsnt1 : Outport  Port=138  OutDataTypeStr=Boolean  PortDimensions=1
  ClimaCfgForSnsrHumPrsnt1_ClimaCfgForSnsrHumPrsnt1 : Outport  Port=139  OutDataTypeStr=Boolean  PortDimensions=1
  ClimaCfgForSnsrPrsntAtRowFirstAtFlrLe_ClimaCfgForSnsrPrsntAtRowFirstAtFlrLe : Outport  Port=140  OutDataTypeStr=Boolean  PortDimensions=1
  ClimaCfgForSnsrPrsntAtRowFirstAtFlrRi_ClimaCfgForSnsrPrsntAtRowFirstAtFlrRi : Outport  Port=141  OutDataTypeStr=Boolean  PortDimensions=1
  ClimaCfgForSnsrPrsntAtRowFirstAtVentSideLe_ClimaCfgForSnsrPrsntAtRowFirstAtVentSideLe : Outport  Port=142  OutDataTypeStr=Boolean  PortDimensions=1
  ClimaCfgForSnsrPrsntAtRowFirstAtVentSideRi_ClimaCfgForSnsrPrsntAtRowFirstAtVentSideRi : Outport  Port=143  OutDataTypeStr=Boolean  PortDimensions=1
  ClimaCfgForSnsrPrsntAtRowSecAtFlrLe_ClimaCfgForSnsrPrsntAtRowSecAtFlrLe : Outport  Port=144  OutDataTypeStr=Boolean  PortDimensions=1
  ClimaCfgForSnsrPrsntAtRowSecAtFlrRi_ClimaCfgForSnsrPrsntAtRowSecAtFlrRi : Outport  Port=145  OutDataTypeStr=Boolean  PortDimensions=1
  ClimaCfgForSnsrPrsntAtRowSecAtVentLe_ClimaCfgForSnsrPrsntAtRowSecAtVentLe : Outport  Port=146  OutDataTypeStr=Boolean  PortDimensions=1
  ClimaCfgForSnsrPrsntAtRowSecAtVentSideLe_ClimaCfgForSnsrPrsntAtRowSecAtVentSideLe : Outport  Port=147  OutDataTypeStr=Boolean  PortDimensions=1
  ClimaCfgForSteerWhlHeatgPrsnt1_ClimaCfgForSteerWhlHeatgPrsnt1 : Outport  Port=148  OutDataTypeStr=Boolean  PortDimensions=1
  ClimaCfgForSteerWhlLeSide1_ClimaCfgForSteerWhlLeSide1 : Outport  Port=149  OutDataTypeStr=Boolean  PortDimensions=1
  ClimaCfgForStrtStp_ClimaCfgForStrtStp : Outport  Port=150  OutDataTypeStr=Enum: CarCfgForStrtStop  PortDimensions=1
  ClimaCfgForThermMgrPrsnt1_ClimaCfgForThermMgrPrsnt1 : Outport  Port=151  OutDataTypeStr=Boolean  PortDimensions=1
  ClimaCfgForVehElec1_ClimaCfgForVehElec1 : Outport  Port=152  OutDataTypeStr=Boolean  PortDimensions=1
  ClimaCfgForVehHev1_ClimaCfgForVehHev1 : Outport  Port=153  OutDataTypeStr=Boolean  PortDimensions=1
  ClimaCfgForVehHyb1_ClimaCfgForVehHyb1 : Outport  Port=154  OutDataTypeStr=Boolean  PortDimensions=1
  ClimaCfgForVehIce1_ClimaCfgForVehIce1 : Outport  Port=155  OutDataTypeStr=Boolean  PortDimensions=1
  ClimaCfgForVehTyp_ClimaCfgForVehTyp : Outport  Port=156  OutDataTypeStr=Enum: CarCfgForVehTyp3  PortDimensions=1
  ClimaCfgForVgaMotorPrsntAtRowFirstLeMid_ClimaCfgForVgaMotorPrsntAtRowFirstLeMid : Outport  Port=157  OutDataTypeStr=Boolean  PortDimensions=1
  ClimaCfgForVgaMotorPrsntAtRowFirstRiMid_ClimaCfgForVgaMotorPrsntAtRowFirstRiMid : Outport  Port=158  OutDataTypeStr=Boolean  PortDimensions=1
  ClimaCfgForVgaMotorPrsntAtRowFirstSideLe_ClimaCfgForVgaMotorPrsntAtRowFirstSideLe : Outport  Port=159  OutDataTypeStr=Boolean  PortDimensions=1
  ClimaCfgForVgaMotorPrsntAtRowFirstSideRi_ClimaCfgForVgaMotorPrsntAtRowFirstSideRi : Outport  Port=160  OutDataTypeStr=Boolean  PortDimensions=1
  ClimaCfgForVgaMotorPrsntAtRowSecSideLe_ClimaCfgForVgaMotorPrsntAtRowSecSideLe : Outport  Port=161  OutDataTypeStr=Boolean  PortDimensions=1
  ClimaCfgForVgaMotorPrsntAtRowSecSideRi_ClimaCfgForVgaMotorPrsntAtRowSecSideRi : Outport  Port=162  OutDataTypeStr=Boolean  PortDimensions=1
  ClimaCfgForVrntOfVehSysElec1_ClimaCfgForVrntOfVehSysElec1 : Outport  Port=163  OutDataTypeStr=Enum: ClimaCenCfgForVrntOfVehSysElec1  PortDimensions=1
  ClimaCfgForWindHeatrPrsnt1_ClimaCfgForWindHeatrPrsnt1 : Outport  Port=164  OutDataTypeStr=Boolean  PortDimensions=1
  ClimaCfgForWtrPmpAuxPrsnt1_ClimaCfgForWtrPmpAuxPrsnt1 : Outport  Port=165  OutDataTypeStr=Boolean  PortDimensions=1
  wires:
  CarCfgForClimaCtrl_CarCfgForAirClng/1 -> ClimaCenCfgMgr_Runnable_sys/1(CarCfgForClimaCtrl_CarCfgForAirClng_read)
  CarCfgForClimaCtrl_CarCfgForAirClnrAdv/1 -> ClimaCenCfgMgr_Runnable_sys/2(CarCfgForClimaCtrl_CarCfgForAirClnrAdv_read)
  CarCfgForClimaCtrl_CarCfgForAirFrag/1 -> ClimaCenCfgMgr_Runnable_sys/3(CarCfgForClimaCtrl_CarCfgForAirFrag_read)
  CarCfgForClimaCtrl_CarCfgForAirMtrlSnsr/1 -> ClimaCenCfgMgr_Runnable_sys/4(CarCfgForClimaCtrl_CarCfgForAirMtrlSnsr_read)
  CarCfgForClimaCtrl_CarCfgForAirQly/1 -> ClimaCenCfgMgr_Runnable_sys/5(CarCfgForClimaCtrl_CarCfgForAirQly_read)
  CarCfgForClimaCtrl_CarCfgForAmntOfSeat/1 -> ClimaCenCfgMgr_Runnable_sys/6(CarCfgForClimaCtrl_CarCfgForAmntOfSeat_read)
  CarCfgForClimaCtrl_CarCfgForAutDemst/1 -> ClimaCenCfgMgr_Runnable_sys/7(CarCfgForClimaCtrl_CarCfgForAutDemst_read)
  CarCfgForClimaCtrl_CarCfgForAuxWtrPmp/1 -> ClimaCenCfgMgr_Runnable_sys/8(CarCfgForClimaCtrl_CarCfgForAuxWtrPmp_read)
  CarCfgForClimaCtrl_CarCfgForBypVlvInHvac/1 -> ClimaCenCfgMgr_Runnable_sys/9(CarCfgForClimaCtrl_CarCfgForBypVlvInHvac_read)
  CarCfgForClimaCtrl_CarCfgForClsVlvInHvac/1 -> ClimaCenCfgMgr_Runnable_sys/10(CarCfgForClimaCtrl_CarCfgForClsVlvInHvac_read)
  CarCfgForClimaCtrl_CarCfgForCmprAcTyp/1 -> ClimaCenCfgMgr_Runnable_sys/11(CarCfgForClimaCtrl_CarCfgForCmprAcTyp_read)
  CarCfgForClimaCtrl_CarCfgForCmptmtClng/1 -> ClimaCenCfgMgr_Runnable_sys/12(CarCfgForClimaCtrl_CarCfgForCmptmtClng_read)
  CarCfgForClimaCtrl_CarCfgForElecVentAdjmt/1 -> ClimaCenCfgMgr_Runnable_sys/13(CarCfgForClimaCtrl_CarCfgForElecVentAdjmt_read)
  CarCfgForClimaCtrl_CarCfgForExtVentnClimaCtrl/1 -> ClimaCenCfgMgr_Runnable_sys/14(CarCfgForClimaCtrl_CarCfgForExtVentnClimaCtrl_read)
  CarCfgForClimaCtrl_CarCfgForFlcTyp/1 -> ClimaCenCfgMgr_Runnable_sys/15(CarCfgForClimaCtrl_CarCfgForFlcTyp_read)
  CarCfgForClimaCtrl_CarCfgForFoldCSD/1 -> ClimaCenCfgMgr_Runnable_sys/16(CarCfgForClimaCtrl_CarCfgForFoldCSD_read)
  CarCfgForClimaCtrl_CarCfgForFuTyp/1 -> ClimaCenCfgMgr_Runnable_sys/17(CarCfgForClimaCtrl_CarCfgForFuTyp_read)
  CarCfgForClimaCtrl_CarCfgForGlassTyp/1 -> ClimaCenCfgMgr_Runnable_sys/18(CarCfgForClimaCtrl_CarCfgForGlassTyp_read)
  CarCfgForClimaCtrl_CarCfgForHeadRadar/1 -> ClimaCenCfgMgr_Runnable_sys/19(CarCfgForClimaCtrl_CarCfgForHeadRadar_read)
  CarCfgForClimaCtrl_CarCfgForHeatPmp/1 -> ClimaCenCfgMgr_Runnable_sys/20(CarCfgForClimaCtrl_CarCfgForHeatPmp_read)
  CarCfgForClimaCtrl_CarCfgForHeatrDurgDrvg/1 -> ClimaCenCfgMgr_Runnable_sys/21(CarCfgForClimaCtrl_CarCfgForHeatrDurgDrvg_read)
  CarCfgForClimaCtrl_CarCfgForHeatrTyp/1 -> ClimaCenCfgMgr_Runnable_sys/22(CarCfgForClimaCtrl_CarCfgForHeatrTyp_read)
  CarCfgForClimaCtrl_CarCfgForHvacFilTyp/1 -> ClimaCenCfgMgr_Runnable_sys/23(CarCfgForClimaCtrl_CarCfgForHvacFilTyp_read)
  CarCfgForClimaCtrl_CarCfgForHvacFlrSnsrForT/1 -> ClimaCenCfgMgr_Runnable_sys/24(CarCfgForClimaCtrl_CarCfgForHvacFlrSnsrForT_read)
  CarCfgForClimaCtrl_CarCfgForHvacHeatrSnsrT/1 -> ClimaCenCfgMgr_Runnable_sys/25(CarCfgForClimaCtrl_CarCfgForHvacHeatrSnsrT_read)
  CarCfgForClimaCtrl_CarCfgForHvacRe/1 -> ClimaCenCfgMgr_Runnable_sys/26(CarCfgForClimaCtrl_CarCfgForHvacRe_read)
  CarCfgForClimaCtrl_CarCfgForHvacTyp/1 -> ClimaCenCfgMgr_Runnable_sys/27(CarCfgForClimaCtrl_CarCfgForHvacTyp_read)
  CarCfgForClimaCtrl_CarCfgForLoVoltElecCfg/1 -> ClimaCenCfgMgr_Runnable_sys/28(CarCfgForClimaCtrl_CarCfgForLoVoltElecCfg_read)
  CarCfgForClimaCtrl_CarCfgForNavSysandIntnmap/1 -> ClimaCenCfgMgr_Runnable_sys/29(CarCfgForClimaCtrl_CarCfgForNavSysandIntnmap_read)
  CarCfgForClimaCtrl_CarCfgForNrOfDoor/1 -> ClimaCenCfgMgr_Runnable_sys/30(CarCfgForClimaCtrl_CarCfgForNrOfDoor_read)
  CarCfgForClimaCtrl_CarCfgForPipeInFuTank/1 -> ClimaCenCfgMgr_Runnable_sys/31(CarCfgForClimaCtrl_CarCfgForPipeInFuTank_read)
  CarCfgForClimaCtrl_CarCfgForPreClng/1 -> ClimaCenCfgMgr_Runnable_sys/32(CarCfgForClimaCtrl_CarCfgForPreClng_read)
  CarCfgForClimaCtrl_CarCfgForPrkgCDSNotAvl/1 -> ClimaCenCfgMgr_Runnable_sys/33(CarCfgForClimaCtrl_CarCfgForPrkgCDSNotAvl_read)
  CarCfgForClimaCtrl_CarCfgForPrkgClima/1 -> ClimaCenCfgMgr_Runnable_sys/34(CarCfgForClimaCtrl_CarCfgForPrkgClima_read)
  CarCfgForClimaCtrl_CarCfgForPrpsnTyp/1 -> ClimaCenCfgMgr_Runnable_sys/35(CarCfgForClimaCtrl_CarCfgForPrpsnTyp_read)